simulink model "s_c856m4kfc"  (R2016b) (continued)
== system s_c856m4kfc == (continued)
      | disp(['Z^-', num2str(latency)]);
  we_delay2 : Subsystem  AncestorBlock=casper_library_delays/pipeline  mask(pipeline){latency=2}
      mask help:
      | An explicitly laid-out delay line to avoid mapping to SRL16s
      | for use in pipelining to help achieve timing closure.
      mask init:
      | pipeline_init(gcb,'latency',latency);
      settings: AttributesFormatString=Latency = %<latency>
      mask Display:
      | port_label('input', 1, 'd');
      | port_label('output', 1, 'q');
      | disp(['Z^-', num2str(latency)]);
  we_delay29 : Subsystem  AncestorBlock=casper_library_delays/pipeline  mask(pipeline){latency=2}
      mask help:
      | An explicitly laid-out delay line to avoid mapping to SRL16s
      | for use in pipelining to help achieve timing closure.
      mask init:
      | pipeline_init(gcb,'latency',latency);
      settings: AttributesFormatString=Latency = %<latency>
      mask Display:
      | port_label('input', 1, 'd');
      | port_label('output', 1, 'q');
      | disp(['Z^-', num2str(latency)]);
  we_delay37 : Subsystem  AncestorBlock=casper_library_delays/pipeline  mask(pipeline){latency=2}
      mask help:
      | An explicitly laid-out delay line to avoid mapping to SRL16s
      | for use in pipelining to help achieve timing closure.
      mask init:
      | pipeline_init(gcb,'latency',latency);
      settings: AttributesFormatString=Latency = %<latency>
      mask Display:
      | port_label('input', 1, 'd');
      | port_label('output', 1, 'q');
      | disp(['Z^-', num2str(latency)]);
  we_delay38 : Subsystem  AncestorBlock=casper_library_delays/pipeline  mask(pipeline){latency=2}
      mask help:
      | An explicitly laid-out delay line to avoid mapping to SRL16s
      | for use in pipelining to help achieve timing closure.
      mask init:
      | pipeline_init(gcb,'latency',latency);
      settings: AttributesFormatString=Latency = %<latency>
      mask Display:
      | port_label('input', 1, 'd');
      | port_label('output', 1, 'q');
      | disp(['Z^-', num2str(latency)]);
  we_delay39 : Subsystem  AncestorBlock=casper_library_delays/pipeline  mask(pipeline){latency=2}
      mask help:
      | An explicitly laid-out delay line to avoid mapping to SRL16s
      | for use in pipelining to help achieve timing closure.
      mask init:
      | pipeline_init(gcb,'latency',latency);
      settings: AttributesFormatString=Latency = %<latency>
      mask Display:
      | port_label('input', 1, 'd');
      | port_label('output', 1, 'q');
      | disp(['Z^-', num2str(latency)]);
  we_delay41 : Subsystem  AncestorBlock=casper_library_delays/pipeline  mask(pipeline){latency=2}
      mask help:
      | An explicitly laid-out delay line to avoid mapping to SRL16s
      | for use in pipelining to help achieve timing closure.
      mask init:
      | pipeline_init(gcb,'latency',latency);
      settings: AttributesFormatString=Latency = %<latency>
      mask Display:
      | port_label('input', 1, 'd');
      | port_label('output', 1, 'q');
      | disp(['Z^-', num2str(latency)]);
  we_delay47 : Subsystem  AncestorBlock=casper_library_delays/pipeline  mask(pipeline){latency=2}
      mask help:
      | An explicitly laid-out delay line to avoid mapping to SRL16s
      | for use in pipelining to help achieve timing closure.
      mask init:
      | pipeline_init(gcb,'latency',latency);
      settings: AttributesFormatString=Latency = %<latency>
      mask Display:
      | port_label('input', 1, 'd');
      | port_label('output', 1, 'q');
      | disp(['Z^-', num2str(latency)]);
  we_delay48 : Subsystem  AncestorBlock=casper_library_delays/pipeline  mask(pipeline){latency=2}
      mask help:
      | An explicitly laid-out delay line to avoid mapping to SRL16s
      | for use in pipelining to help achieve timing closure.
      mask init:
      | pipeline_init(gcb,'latency',latency);
      settings: AttributesFormatString=Latency = %<latency>
      mask Display:
      | port_label('input', 1, 'd');
      | port_label('output', 1, 'q');
      | disp(['Z^-', num2str(latency)]);
  we_delay49 : Subsystem  AncestorBlock=casper_library_delays/pipeline  mask(pipeline){latency=2}
      mask help:
      | An explicitly laid-out delay line to avoid mapping to SRL16s
      | for use in pipelining to help achieve timing closure.
      mask init:
      | pipeline_init(gcb,'latency',latency);
      settings: AttributesFormatString=Latency = %<latency>
      mask Display:
      | port_label('input', 1, 'd');
      | port_label('output', 1, 'q');
      | disp(['Z^-', num2str(latency)]);
  we_delay50 : Subsystem  AncestorBlock=casper_library_delays/pipeline  mask(pipeline){latency=2}
      mask help:
      | An explicitly laid-out delay line to avoid mapping to SRL16s
      | for use in pipelining to help achieve timing closure.
      mask init:
      | pipeline_init(gcb,'latency',latency);
      settings: AttributesFormatString=Latency = %<latency>
      mask Display:
      | port_label('input', 1, 'd');
      | port_label('output', 1, 'q');
      | disp(['Z^-', num2str(latency)]);
  we_delay7 : Subsystem  AncestorBlock=casper_library_delays/pipeline  mask(pipeline){latency=2}
      mask help:
      | An explicitly laid-out delay line to avoid mapping to SRL16s
      | for use in pipelining to help achieve timing closure.
      mask init:
      | pipeline_init(gcb,'latency',latency);
      settings: AttributesFormatString=Latency = %<latency>
      mask Display:
      | port_label('input', 1, 'd');
      | port_label('output', 1, 'q');
      | disp(['Z^-', num2str(latency)]);
  x_setup : Subsystem  Tag=xps:sw_reg  AncestorBlock=xps_library/software_register  mask(swreg){io_dir=From Processor, io_delay=0, sample_period=1, names=[f_per_x ip_per_x num_x snap_arm pktstart_mux pack_mux pktoffset_sel], bitwidths=[15 4 9 1 1 1 1], bin_pts=[0 2 0 0 0 0 0], arith_types=[0 0 0 2 0 2 0], sim_port=off, show_format=on}
      mask prompts: io_dir "I/O direction"; io_delay "I/O delay"; sample_period "Sample period"; names "Bitfield names [msb...lsb]"; bitwidths "Bitfield widths"; bin_pts "Bitfield binary pts"; arith_types "Bitfield types, ufix=0, fix=1, bool=2"; sim_port "Provide sim input/output?"; show_format "Print format string?"
      mask help: A 32-bit software-accessible register. Can be divided into bitfields of varying widths and types using the fields in the block mask.
      mask init:
      | swreg_init(gcb)
      settings: AttributesFormatString=7 inputs:uf15.0,uf4.2,uf9.0,b,uf1.0,b,uf1.0 = 32 bits
      mask TypeOptions io_dir: To Processor | From Processor
      mask Callback io_dir:
      | mask_enables = get_param(gcb, 'MaskEnables');
      | mask_names = get_param(gcb, 'MaskNames');
      | if strcmp(get_param(gcb, 'io_dir'), 'To Processor'),
      |     mask_enables{ismember(mask_names, 'sample_period')} = 'off';
      | else
      |     mask_enables{ismember(mask_names, 'sample_period')} = 'on';
      | end
      | set_param(gcb, 'MaskEnables', mask_enables);
      mask DialogControl ParameterTabVar0 Prompt: Setup
  xsg_core_config : Subsystem  Tag=xps:xsg  AncestorBlock=xps_library/XSG_core_config  mask(xsg core config){hw_sys=SKARAB:xc7vx690t, clk_src=sys_clk, clk_rate=238, sample_period=1, synthesis_tool=XST}
      mask prompts: hw_sys "Hardware Platform"; clk_src "User IP Clock source"; clk_rate "User IP Clock Rate (MHz)"; sample_period "Sample Period"; synthesis_tool "Synthesis Tool:"
      mask help:
      | The XSG Core Config block is used to configure the System Generator design
      | for the casper_xps toolflow. Settings here are used to configure the
      | Xilinx System Generator block parameters automatically, and control toolflow
      | script  execution. It needs to be at the top level of all designs being compiled
      | with the casper_xps toolflow.
      mask init:
      | xps_xsg_conf_mask;
      mask Help: eval('xlWeb([getenv(''MLIB_ROOT''), ''\doc\html\sys_xsg_core_config\sys_xsg_core_config.html''])')
      mask TypeOptions hw_sys: ROACH:sx95t | ROACH:lx110t | ROACH2:sx475t | SNAP:xc7k160t | KC705:xc7k325t | SKARAB:xc7vx690t
      mask Callback hw_sys: xps_xsg_conf_callback
      mask TypeOptions clk_src: sys_clk | aux_clk
      mask Callback clk_src: xps_xsg_conf_callback
      mask Callback clk_rate: xps_xsg_conf_callback
      mask TypeOptions synthesis_tool: Leonardo Spectrum | Synplify | Synplify Pro | XST
      mask Display: fprintf(['MSSGE\n', hw_sys]);
  tag scope: acc_scale=global, cnt_rst=global, ct_board_hmc_offset=global, ct_chans_per_board=global, ct_chans_per_x=global, ct_chans_per_x_hmc_par=global, ct_chans_per_x_hmc_par_recip=global, ct_chans_per_x_par=global, ct_chans_per_x_recip=global, ct_fake_data_error=global, ct_freq_gen_offset=global, ct_num_x_boards=global, ct_num_x_boards_recip=global, ct_num_xeng=global, ct_num_xeng_recip=global, ct_rst_err_latch=global, ct_tag_insert_sel=global, ct_tvg_en2=global, ct_tvg_en=global, ct_x_per_board=global, ct_x_per_board_recip=global, force_gbe_txen=global, obuf_read_gap=global, pfb_bypass=global, pktoffset_sel=global, pktstart_sel=global, sys_rst=global, ts_tvg_en=global, tvgtone_levels=global, tvgtone_p0=global, tvgtone_p1=global, up_timestep_error=global
  wires:
  Constant3/1 -> gbe0/8(rx_overrun_ack)
  Constant4/1 -> gbe0/7(rx_ack)
  [gbe_rst] -> pipeline99/1(d)
  unpack_and_reorder/5(recvd) -> ts_tvg/6(recvd_in)
  sync_gen1/2(dout) -> bus_expand6/1(bus_in)
  sync_gen1/3(dvalid) -> impulse_gen0/6(en), impulse_gen1/6(en)
  Slice4/1 -> pipeline16/1(d)
  pipeline16/1(q) -> local_time_lsw/1(out_timestamp_lsw)
  Slice16/1 -> Convert6/1
  pipeline47/1(q) -> local_time_msw/1(out_timestamp_msw)
  Convert6/1 -> pipeline47/1(d)
  [time80_48] -> Slice16/1, Slice4/1
  Slice12/1 -> [time80]
  impulse_gen0/3(dvalid) -> [valid80]
  impulse_gen0/1(sync_out) -> [sync80]
  impulse_gen0/2(dout) -> [d80_adc0]
  impulse_gen0/4(misco) -> [time80_48], Slice12/1
  [tvg_adc0_en] -> impulse_gen0/1(tvg_en)
  impulse_gen1/1(sync_out) -> Terminator6/1
  impulse_gen1/2(dout) -> [d80_adc1]
  impulse_gen1/3(dvalid) -> Terminator8/1
  impulse_gen1/4(misco) -> [dig_flags]
  [tvg_adc1_en] -> impulse_gen1/1(tvg_en)
  impulse0/1(in_offset) -> Slice7/1
  impulse0/2(in_amplitude) -> impulse_gen0/3(impulse_amplitude)
  impulse1/1(in_offset) -> Slice8/1
  impulse1/2(in_amplitude) -> impulse_gen1/3(impulse_amplitude)
  sync_gen1/1(sync) -> impulse_gen0/4(sync_in), impulse_gen1/4(sync_in)
  bus_expand6/1(msb_out2) -> impulse_gen0/5(din)
  bus_expand6/2(lsb_out1) -> impulse_gen1/5(din)
  sync_gen1/5(misco) -> impulse_gen1/7(misci)
  sync_gen1/4(time_out) -> impulse_gen0/7(misci)
  Constant31/1 -> Concat9/2
  [unpack_timestamp] -> Concat9/1
  [up_timestep_error] -> Logical15/1
  [sys_rst] -> Logical15/2
  Logical15/1 -> edge_detect17/1(in)
  edge_detect17/1(out) -> [sys_relock]
  Concat9/1 -> sync_gen1/1(time_in)
  [sys_relock] -> sync_gen1/5(relock)
  [unpack_dv] -> sync_gen1/2(en)
  [unpack_d160] -> sync_gen1/3(din)
  Slice7/1 -> impulse_gen0/2(impulse_offset)
  Slice8/1 -> impulse_gen1/2(impulse_offset)
  trig_time_msw/1(in_msw) -> pipeline28/1(d)
  trig_time_lsw/1(in_lsw) -> pipeline44/1(d)
  Concat13/1 -> Relational10/1
  pipeline28/1(q) -> Concat13/1
  pipeline44/1(q) -> Concat13/2
  [valid80] -> Counter/2
  [sync80] -> Counter/1
  Constant12/1 -> Relational7/2
  Counter/1 -> Relational7/1
  Relational7/1 -> Mux/3
  [adc_snap_arm] -> Logical14/2
  [time80_48] -> Relational10/2
  [adc_snap_trig_source] -> Mux/1
  edge_detect4/1(out) -> pipeline5/1(d)
  control/7(in_cnt_rst) -> edge_detect1/1(in)
  edge_detect1/1(out) -> pipeline14/1(d)
  control/3(in_gbe_rst) -> pipeline6/1(d)
  control/1(in_sys_rst) -> pipeline3/1(d)
  pipeline3/1(q) -> [sys_rst]
  pipeline5/1(q) -> [clr_status]
  pipeline6/1(q) -> [gbe_rst]
  pipeline7/1(q) -> [gbe_txen]
  pipeline13/1(q) -> [tvg_adc0_en]
  pipeline14/1(q) -> [cnt_rst]
  control/5(in_tvg_adc0) -> pipeline13/1(d)
  edge_detect2/1(out) -> pipeline38/1(d)
  pipeline38/1(q) -> [gbe_cnt_rst]
  control/8(in_gbe_cnt_rst) -> edge_detect2/1(in)
  control/4(in_gbe_txen) -> pipeline7/1(d)
  control/2(in_status_clr) -> edge_detect4/1(in)
  pipeline43/1(q) -> [adc_snap_arm]
  control/9(in_adc_snap_arm) -> pipeline43/1(d)
  control/10(in_adc_snap_trig_select) -> pipeline39/1(d)
  pipeline39/1(q) -> [adc_snap_trig_source]
  pfb/2(dout0) -> [pfb_d200_0]
  pfb/3(dout1) -> [pfb_d200_1]
  fft_shift/1(in_fft_shift) -> pipeline4/1(d)
  pfb/5(of) -> [pfb_of]
  pfb/4(dvalid) -> [pfb_d200_dv]
  pfb/1(sync_out) -> [pfb_d200_sync]
  tl_fd0/2(enable) -> phase_compensation0/5(load_value)
  tl_fd1/1(value) -> phase_compensation1/4(delay_value)
  tl_fd1/2(enable) -> phase_compensation1/5(load_value)
  tl_fd0/1(value) -> phase_compensation0/4(delay_value)
  [clr_status] -> tl_fd1/1(rst), tl_fd0/1(rst)
  phase_compensation0/1(sync_out) -> [fdfs_sync]
  phase_compensation1/1(sync_out) -> Terminator24/1
  phase_compensation0/2(dout) -> [fdfs_d144_0]
  phase_compensation1/2(dout) -> [fdfs_d144_1]
  phase_compensation0/3(dvalid) -> [fdfs_dv]
  phase_compensation1/3(dvalid) -> Terminator25/1
  [ld_val0] -> tl_fd0/3(load_value)
  [ld_val1] -> tl_fd1/3(load_value)
  bus_expand16/1(msb_out2) -> tl_fd0/5(din)
  tl_fd0/5(dvalid) -> phase_compensation0/3(en)
  tl_fd0/3(sync_out) -> phase_compensation0/1(sync_in)
  tl_fd0/4(dout) -> phase_compensation0/2(din)
  tl_fd1/4(dout) -> phase_compensation1/2(din)
  bus_expand16/2(lsb_out1) -> tl_fd1/5(din)
  Constant29/1 -> Concat2/2
  Concat2/1 -> tl_fd1/2(current_time), tl_fd0/2(current_time)
  [gbe_cnt_rst] -> pipeline31/1(d)
  pipeline31/1(q) -> gbe0/9(debug_rst)
  Counter2/1 -> pipeline29/1(d)
  Constant26/1 -> eq1/2(data_in)
  Constant11/1 -> eq1/3(we)
  Delay53/1 -> quant1/1(sync_in)
  Delay34/1 -> quant1/2(din)
  eq1/1(data_out) -> quant1/4(coeffs)
  Delay50/1 -> quant1/3(en)
  Counter4/1 -> pipeline27/1(d)
  Constant24/1 -> eq0/2(data_in)
  Constant25/1 -> eq0/3(we)
  Delay51/1 -> quant0/1(sync_in)
  Delay2/1 -> quant0/2(din)
  Delay52/1 -> quant0/3(en)
  eq0/1(data_out) -> quant0/4(coeffs)
  quant0/1(sync_out) -> [q_sync]
  quant0/2(dout) -> [q_d64_0]
  quant0/3(dvalid) -> [q_dv]
  quant0/4(of) -> [q_of0]
  quant1/2(dout) -> [q_d64_1]
  quant1/1(sync_out) -> Terminator19/1
  quant1/3(dvalid) -> Terminator21/1
  quant1/4(of) -> [q_of1]
  pipeline27/1(q) -> eq0/1(addr)
  pipeline29/1(q) -> eq1/1(addr)
  [fdfs_sync] -> Delay51/1, pipeline42/1(d)
  [fdfs_d144_0] -> Delay2/1
  [fdfs_dv] -> Delay52/1, pipeline41/1(d)
  pipeline41/1(q) -> Counter4/2
  pipeline42/1(q) -> Counter4/1
  pipeline45/1(q) -> Counter2/2
  [fdfs_sync] -> Delay53/1, pipeline48/1(d)
  [fdfs_d144_1] -> Delay34/1
  [fdfs_dv] -> Delay50/1, pipeline45/1(d)
  pipeline48/1(q) -> Counter2/1
  gbe0/13(rx_dest_ip) -> pipeline115/1(d)
  gbe0/14(rx_dest_port) -> pipeline116/1(d)
  [rx_dest_ip] -> pipeline/1(d)
  pipeline/1(q) -> rx_dest_ip/1(out_reg)
  gbe0/1(led_up) -> pipeline100/1(d)
  [forty_gbe_led_up] -> pipeline19/1(d)
  gbe0/2(led_rx) -> pipeline101/1(d)
  [forty_gbe_led_rx] -> pipeline20/1(d)
  gbe0/3(led_tx) -> pipeline102/1(d)
  [forty_gbe_led_tx] -> pipeline51/1(d)
  gbe0/4(tx_afull) -> pipeline103/1(d)
  gbe0/5(tx_overflow) -> pipeline104/1(d)
  gbe0/12(rx_overrun) -> pipeline114/1(d)
  pipeline19/1(q) -> gbe_status/1(out_led_up)
  pipeline20/1(q) -> gbe_status/2(out_led_rx)
  pipeline51/1(q) -> gbe_status/3(out_led_tx)
  gbe0/8(rx_source_ip) -> pipeline110/1(d)
  gbe0/9(rx_source_port) -> pipeline111/1(d)
  gbe0/6(rx_data) -> pipeline108/1(d)
  gbe0/10(rx_end_of_frame) -> pipeline112/1(d)
  gbe0/11(rx_bad_frame) -> pipeline113/1(d)
  pipeline80/1(q) -> [flag_sync]
  pipeline81/1(q) -> [flag_misc]
  pipeline82/1(q) -> [flag_dvalid]
  pipeline83/1(q) -> [flag_time]
  pipeline85/1(q) -> time_gen1/4(rd_sync_in)
  pipeline87/1(q) -> time_gen1/6(rd_misc_in)
  [s256_d] -> pipeline98/1(d)
  [s256_ip] -> pipeline97/1(d)
  [s256_port] -> pipeline96/1(d)
  Logical2/1 -> gbe0/6(tx_end_of_frame)
  [s256_v] -> Logical1/2
  [s256_eof] -> Logical2/2
  Delay1/1 -> Logical1/1, Logical2/1
  [s256_eof] -> Delay1/2
  pipeline96/1(q) -> gbe0/5(tx_dest_port)
  pipeline97/1(q) -> gbe0/4(tx_dest_ip)
  pipeline98/1(q) -> gbe0/2(tx_data)
  pipeline99/1(q) -> gbe0/1(rst)
  Logical4/1 -> Delay1/1
  pipeline100/1(q) -> [forty_gbe_led_up]
  pipeline101/1(q) -> [forty_gbe_led_rx]
  pipeline102/1(q) -> [forty_gbe_led_tx]
  pipeline103/1(q) -> [forty_gbe_tx_full]
  pipeline104/1(q) -> [forty_gbe_tx_of]
  pipeline108/1(q) -> [forty_gbe_rx_data]
  pipeline110/1(q) -> [forty_gbe_rx_src_ip]
  pipeline111/1(q) -> [forty_gbe_rx_src_port]
  pipeline112/1(q) -> [forty_gbe_rx_eof]
  pipeline113/1(q) -> [forty_gbe_rx_bad]
  pipeline114/1(q) -> [forty_gbe_rx_overrun]
  pipeline115/1(q) -> [rx_dest_ip]
  pipeline116/1(q) -> [rx_dest_port]
  [forty_gbe_rx_data] -> unpack_and_reorder/1(d256)
  [forty_gbe_rx_valid] -> unpack_and_reorder/2(dv256)
  [forty_gbe_rx_eof] -> unpack_and_reorder/3(eof256)
  unpack_and_reorder/2(d160) -> ts_tvg/3(data_in)
  unpack_and_reorder/3(dv) -> ts_tvg/4(dv_in)
  unpack_and_reorder/4(time) -> ts_tvg/5(time_in)
  unpack_and_reorder/1(sync) -> ts_tvg/2(sync_in)
  pipeline10/1(q) -> [unpack_sync]
  pipeline11/1(q) -> [unpack_d160]
  pipeline12/1(q) -> [unpack_dv]
  pipeline15/1(q) -> [unpack_timestamp]
  [clr_status] -> Subsystem5/1(rst)
  Delay/1 -> pipeline85/1(d)  "gapsync"
  Delay3/1 -> pipeline86/1(d)  "gapdata"
  Mux1/1 -> pipeline87/1(d)  "gapvalid"
  tl_fd0/7(misco) -> Terminator2/1
  tl_fd0/6(time) -> Terminator3/1
  tl_fd1/6(time) -> Terminator4/1
  tl_fd1/3(sync_out) -> phase_compensation1/1(sync_in)
  tl_fd1/5(dvalid) -> phase_compensation1/3(en)
  tl_fd1/7(misco) -> Terminator5/1
  Constant18/1 -> tl_fd0/7(misci)
  Constant19/1 -> tl_fd1/7(misci)
  Constant1/1 -> sync_gen1/4(misci)
  time_gen/1(rd_sync_out) -> tl_fd1/4(sync_in), tl_fd0/4(sync_in)
  time_gen/2(rd_dvalid) -> tl_fd1/6(en), tl_fd0/6(en)
  time_gen/3(rd_val_out) -> Concat2/1
  time_gen/4(rd_misc_out) -> bus_expand16/1(bus_in)
  [sync80] -> time_gen/1(wr_sync_in)
  [valid80] -> time_gen/2(wr_en)
  [pfb_sync] -> time_gen/4(rd_sync_in)
  [pfb_dv] -> time_gen/5(rd_en)
  [pfb_d144_1] -> Concat1/2
  [pfb_d144_0] -> Concat1/1
  Concat1/1 -> time_gen/6(rd_misc_in)
  [time80] -> Slice2/1
  pipeline86/1(q) -> time_gen1/5(rd_en)
  time_gen1/1(rd_sync_out) -> pipeline80/1(d)
  time_gen1/4(rd_misc_out) -> pipeline81/1(d)
  time_gen1/2(rd_dvalid) -> pipeline82/1(d)
  time_gen1/3(rd_val_out) -> pipeline83/1(d)
  [time80] -> time_gen/3(wr_val_in)
  Slice2/1 -> time_gen1/3(wr_val_in)
  pipeline4/1(q) -> [fft_shift]
  Logical1/1 -> gbe0/3(tx_valid)
  pipeline59/1(q) -> [ct_tvg_en]
  control/11(in_ct_tvg_en) -> pipeline59/1(d)
  pipeline52/1(q) -> [up_dbg_rst]
  control/12(in_unpack_debug_reset) -> pipeline52/1(d)
  [clr_status] -> Subsystem3/1(rst)
  Slice/1 -> Subsystem3/2(in0)
  [pfb_of] -> Slice/1, Slice6/1
  Slice6/1 -> Subsystem3/3(in1)
  [pfb_sync] -> Subsystem3/4(in2)
  Subsystem3/3(out2) -> pfb_status/3(out_sync_cnt)
  Subsystem5/1(out0) -> quant_status/1(out_pol0_or_cnt)
  Relational/1 -> Subsystem5/2(in0)
  Constant10/1 -> Relational/2
  [q_of0] -> Relational/1
  Relational3/1 -> Subsystem5/3(in1)
  [q_of1] -> Relational3/1
  Constant13/1 -> Relational3/2
  Subsystem5/2(out1) -> quant_status/2(out_pol1_or_cnt)
  Subsystem3/1(out0) -> pfb_status/1(out_pol0_or_err_cnt)
  Subsystem3/2(out1) -> pfb_status/2(out_pol1_or_err_cnt)
  [ct_tvg_en2] -> Mux1/1
  Delay17/1 -> Delay5/1
  Delay6/1 -> Mux1/2
  Delay7/1 -> tvg/1(sync), Delay/1
  Delay18/1 -> Delay7/1
  Delay16/1 -> Delay6/1
  Delay5/1 -> tvg/2(dv), Delay3/1
  tvg/1(data256) -> Mux1/3
  [q_dv] -> qt_block_check/1(dv)
  [cnt_rst] -> Subsystem12/1(rst)
  Subsystem12/1(out0) -> qt_dv_err/1(out_dvblock_err_cnt)
  qt_block_check/1(error) -> Subsystem12/2(in0)
  [cd_bypass] -> Terminator28/1
  [cd_bypass] -> Terminator26/1
  [delay_sync] -> [pfbin_sync]
  [delay_dv] -> [pfbin_dv]
  [cd_bypass] -> Terminator22/1
  [delay_d0] -> [pfbin_d0]
  [cd_bypass] -> Terminator18/1
  [delay_d1] -> [pfbin_d1]
  control/13(in_cd_bypass) -> pipeline131/1(d)
  pipeline131/1(q) -> [cd_bypass]
  Concat22/1 -> [ld_val0]
  Concat3/1 -> [ld_val1]
  phase0/1(in_initial) -> Reinterpret2/1
  delta_delay0/1(in_delta) -> Reinterpret1/1
  phase0/2(in_delta) -> Reinterpret3/1
  Reinterpret8/1 -> Concat22/1
  Reinterpret1/1 -> Concat22/2
  Reinterpret2/1 -> Concat22/3
  Reinterpret3/1 -> Concat22/4
  phase1/1(in_initial) -> Reinterpret5/1
  delta_delay1/1(in_delta) -> Reinterpret4/1
  phase1/2(in_delta) -> Reinterpret6/1
  Reinterpret4/1 -> Concat3/2
  Reinterpret5/1 -> Concat3/3
  Reinterpret6/1 -> Concat3/4
  Reinterpret7/1 -> Concat3/1
  delay0/1(in_initial) -> Reinterpret8/1
  delay1/1(in_initial) -> Reinterpret7/1
  [clr_status] -> Subsystem2/1(rst)
  [cd_error] -> Subsystem2/2(in0)
  [flag_dvalid] -> Subsystem13/1(In1)
  Subsystem13/1(Out1) -> pack_in_dv_rate/1(out_dv_rate)
  [s256_v] -> Subsystem11/1(In1)
  Subsystem11/1(Out1) -> pack_out_dv_rate/1(out_dv_rate)
  pipeline121/1(q) -> Convert/1
  pipeline124/1(q) -> [ip_per_x]
  pipeline125/1(q) -> [num_x]
  x_setup/1(in_f_per_x) -> pipeline121/1(d)
  x_setup/2(in_ip_per_x) -> pipeline124/1(d)
  x_setup/3(in_num_x) -> pipeline125/1(d)
  pipeline120/1(q) -> [pack_snap_arm]
  x_setup/4(in_snap_arm) -> pipeline120/1(d)
  pipeline130/1(q) -> [pktstart_sel]
  x_setup/5(in_pktstart_mux) -> pipeline130/1(d)
  [s256_v] -> pack_block_check/1(dv)
  [cnt_rst] -> Subsystem14/1(rst)
  Subsystem14/1(out0) -> pack_dv_err/1(out_dvblock_err_cnt)
  pack_block_check/1(error) -> [pack_dv_err], Subsystem14/2(in0)
  pipeline149/1(q) -> [pack_mux]
  x_setup/6(in_pack_mux) -> pipeline149/1(d)
  tl_cd0_control0/3(in_load_time_msw) -> Concat6/1
  tl_cd0_control/1(in_load_time_lsw) -> Concat6/2
  Constant42/1 -> tl_cd0_control/1(sim_1)
  Constant40/1 -> tl_cd0_control0/3(sim_3)
  Constant41/1 -> tl_cd0_control0/2(sim_2)
  Constant43/1 -> Delay12/1
  Delay12/1 -> tl_cd0_control0/1(sim_1)
  Concat6/1 -> pipeline136/1(d)
  tl_cd0_control0/2(in_load_immediate) -> pipeline135/1(d)
  tl_cd0_control0/1(in_arm) -> pipeline134/1(d)
  tl_cd1_control0/3(in_load_time_msw) -> Concat8/1
  tl_cd1_control/1(in_load_time_lsw) -> Concat8/2
  Constant47/1 -> tl_cd1_control/1(sim_1)
  Constant45/1 -> tl_cd1_control0/3(sim_3)
  Constant46/1 -> tl_cd1_control0/2(sim_2)
  Constant48/1 -> Delay13/1
  Delay13/1 -> tl_cd1_control0/1(sim_1)
  Concat8/1 -> pipeline143/1(d)
  tl_cd1_control0/2(in_load_immediate) -> pipeline138/1(d)
  tl_cd1_control0/1(in_arm) -> pipeline137/1(d)
  [ld_val0] -> pipeline73/1(d)
  [clr_status] -> pipeline25/1(d), pipeline75/1(d)
  tl_cd0/6(time) -> [time80_tl_cd0]
  Constant6/1 -> Concat15/2
  Concat15/1 -> pipeline24/1(d), Gateway Out7/1, pipeline74/1(d)
  [time80] -> Concat15/1
  pipeline75/1(q) -> tl_cd0/1(rst)
  pipeline74/1(q) -> tl_cd0/2(current_time)
  pipeline73/1(q) -> tl_cd0/3(load_value)
  pipeline72/1(q) -> tl_cd0/4(sync_in)
  pipeline71/1(q) -> tl_cd0/5(din)
  pipeline76/1(q) -> tl_cd0/6(en)
  [sync80] -> pipeline36/1(d), pipeline72/1(d)
  [valid80] -> pipeline34/1(d), pipeline76/1(d)
  Constant16/1 -> tl_cd0/7(misci)
  tl_cd0/7(misco) -> Terminator/1
  tl_cd0/3(sync_out) -> [sync_tl_cd0]
  tl_cd0/5(dvalid) -> [dvalid_tl_cd0]
  tl_cd0/4(dout) -> [dout_tl_cd0]
  tl_cd0/2(enable) -> [en_tl_cd0]
  tl_cd0/1(value) -> [delay_tl_cd0]
  pipeline92/1(q) -> tl_cd0/10(load_time)
  pipeline91/1(q) -> tl_cd0/9(load_immediate)
  pipeline90/1(q) -> tl_cd0/8(arm)
  [tl_cd0_arm] -> pipeline90/1(d)
  [tl_cd0_load_immediate] -> pipeline91/1(d)
  [tl_cd0_load_time] -> pipeline92/1(d)
  Gateway Out7/1 -> To Workspace7/1
  [d80_adc0] -> pipeline71/1(d)
  [dvalid_tl_cd0] -> Delay22/1
  [sync_tl_cd0] -> Delay21/1
  [en_tl_cd0] -> Delay24/1
  [delay_tl_cd0] -> Delay23/1
  Concat5/1 -> time_gen_cd/6(rd_misc_in)
  time_gen_cd/4(rd_misc_out) -> bus_expand2/1(bus_in)
  [sync_tl_cd0] -> time_gen_cd/1(wr_sync_in)
  [dvalid_tl_cd0] -> time_gen_cd/2(wr_en)
  [hmc_sync_p0] -> time_gen_cd/4(rd_sync_in)
  [hmc_dvalid] -> time_gen_cd/5(rd_en)
  time_gen_cd/1(rd_sync_out) -> [tg_rd_sync]
  time_gen_cd/2(rd_dvalid) -> [tg_rd_dvalid]
  [dvalid_tl_bs0] -> wb_prog_delay/4(en)
  [delay_tl_bs0] -> wb_prog_delay/2(delay_val)
  [delay_tl_sync0] -> wb_prog_delay/1(sync)
  [en_tl_bs0] -> wb_prog_delay/5(load_val)
  wb_prog_delay/2(data_out) -> [delay_d0]
  wb_prog_delay/3(dvalid) -> [delay_dv]
  [time80_tl_cd0] -> Slice9/1
  Slice9/1 -> time_gen_cd/3(wr_val_in)
  Constant35/1 -> Concat7/2
  time_gen_cd/3(rd_val_out) -> Concat7/1
  Concat7/1 -> [tg_rd_time_out]
  wb_prog_delay/1(sync_out) -> [delay_sync]
  pipeline77/1(q) -> tl_cd_bs0/1(rst)
  pipeline1/1(q) -> tl_cd_bs0/2(current_time)
  pipeline78/1(q) -> tl_cd_bs0/3(load_value)
  pipeline84/1(q) -> tl_cd_bs0/4(sync_in)
  pipeline88/1(q) -> tl_cd_bs0/5(din)
  pipeline89/1(q) -> tl_cd_bs0/6(en)
  [clr_status] -> pipeline77/1(d)
  [tg_rd_time_out] -> pipeline1/1(d)
  [ld_val0] -> pipeline78/1(d)
  [tg_rd_sync] -> pipeline84/1(d)
  [tg_rd_dvalid] -> pipeline89/1(d)
  Constant34/1 -> tl_cd_bs0/7(misci)
  tl_cd_bs0/7(misco) -> Terminator12/1
  tl_cd_bs0/4(dout) -> [data_tl_bs0]
  tl_cd_bs0/6(time) -> Terminator13/1
  tl_cd_bs0/5(dvalid) -> [dvalid_tl_bs0]
  tl_cd_bs0/1(value) -> [delay_tl_bs0]
  tl_cd_bs0/3(sync_out) -> [delay_tl_sync0]
  tl_cd_bs0/2(enable) -> [en_tl_bs0]
  pipeline21/1(q) -> tl_cd_bs0/8(arm)
  pipeline22/1(q) -> tl_cd_bs0/9(load_immediate)
  pipeline23/1(q) -> tl_cd_bs0/10(load_time)
  [tl_cd0_arm] -> pipeline21/1(d)
  [tl_cd0_load_immediate] -> pipeline22/1(d)
  [tl_cd0_load_time] -> pipeline23/1(d)
  [data_tl_bs0] -> wb_prog_delay/3(din)
  [tg_data_p0] -> pipeline88/1(d)
  pipeline25/1(q) -> tl_cd1/1(rst)
  [ld_val1] -> pipeline26/1(d)
  pipeline24/1(q) -> tl_cd1/2(current_time)
  pipeline26/1(q) -> tl_cd1/3(load_value)
  pipeline36/1(q) -> tl_cd1/4(sync_in)
  pipeline33/1(q) -> tl_cd1/5(din)
  pipeline34/1(q) -> tl_cd1/6(en)
  pipeline37/1(q) -> tl_cd1/9(load_immediate)
  pipeline35/1(q) -> tl_cd1/8(arm)
  pipeline40/1(q) -> tl_cd1/10(load_time)
  [tl_cd1_arm] -> pipeline35/1(d)
  [tl_cd1_load_immediate] -> pipeline37/1(d)
  [tl_cd1_load_time] -> pipeline40/1(d)
  Constant17/1 -> tl_cd1/7(misci)
  tl_cd1/1(value) -> [delay_tl_cd1]
  tl_cd1/2(enable) -> [en_tl_cd1]
  tl_cd1/3(sync_out) -> [sync_tl_cd1]
  tl_cd1/4(dout) -> [dout_tl_cd1]
  tl_cd1/5(dvalid) -> [dvalid_tl_cd1]
  tl_cd1/6(time) -> [time80_tl_cd1]
  tl_cd1/7(misco) -> Terminator1/1
  [delay_tl_cd1] -> Delay27/1
  [en_tl_cd1] -> Delay28/1
  [sync_tl_cd1] -> Delay25/1
  [dvalid_tl_cd1] -> Delay26/1
  pipeline54/1(q) -> tl_cd_bs1/1(rst)
  pipeline53/1(q) -> tl_cd_bs1/2(current_time)
  pipeline60/1(q) -> tl_cd_bs1/3(load_value)
  pipeline61/1(q) -> tl_cd_bs1/4(sync_in)
  pipeline67/1(q) -> tl_cd_bs1/5(din)
  pipeline68/1(q) -> tl_cd_bs1/6(en)
  [clr_status] -> pipeline54/1(d)
  [tg_rd_time_out] -> pipeline53/1(d)
  [ld_val1] -> pipeline60/1(d)
  [tg_rd_sync] -> pipeline61/1(d)
  [tg_rd_dvalid] -> pipeline68/1(d)
  [tg_data_p1] -> pipeline67/1(d)
  Constant36/1 -> tl_cd_bs1/7(misci)
  pipeline156/1(q) -> tl_cd_bs1/8(arm)
  pipeline69/1(q) -> tl_cd_bs1/9(load_immediate)
  pipeline70/1(q) -> tl_cd_bs1/10(load_time)
  [tl_cd1_load_time] -> pipeline70/1(d)
  [tl_cd1_load_immediate] -> pipeline69/1(d)
  [tl_cd1_arm] -> pipeline156/1(d)
  tl_cd_bs1/7(misco) -> Terminator10/1
  tl_cd_bs1/4(dout) -> [data_tl_bs1]
  tl_cd_bs1/6(time) -> Terminator7/1
  tl_cd_bs1/5(dvalid) -> [dvalid_tl_bs1]
  tl_cd_bs1/1(value) -> [delay_tl_bs1]
  tl_cd_bs1/3(sync_out) -> [delay_tl_sync1]
  tl_cd_bs1/2(enable) -> [en_tl_bs1]
  [delay_tl_sync1] -> wb_prog_delay1/1(sync)
  [delay_tl_bs1] -> wb_prog_delay1/2(delay_val)
  [en_tl_bs1] -> wb_prog_delay1/5(load_val)
  [data_tl_bs1] -> wb_prog_delay1/3(din)
  [dvalid_tl_bs1] -> wb_prog_delay1/4(en)
  wb_prog_delay1/2(data_out) -> [delay_d1]
  wb_prog_delay1/1(sync_out) -> Terminator16/1
  wb_prog_delay1/3(dvalid) -> Terminator11/1
  [d80_adc1] -> pipeline33/1(d)
  Subsystem7/1(per_sec) -> reorder_status1/1(out_dv_per_sec)
  [upg_reo_time] -> Subsystem1/3(time)
  [upg_reo_recv] -> Subsystem1/4(recv)
  [upg_reo_dv] -> Subsystem7/1(dv), Subsystem1/2(dv)
  [cnt_rst] -> Subsystem1/1(rst)
  Subsystem1/7(time_change) -> [reo_time_change]
  Logical5/1 -> Subsystem1/7(overflow_err)
  [upg_of80_p1] -> Logical5/2
  [upg_of80_p0] -> Logical5/1
  Subsystem1/8(timestep_err) -> [up_timestep_error]
  [upg_reo_disc] -> Subsystem1/5(discard_err)
  [upg_reo_relock] -> Subsystem1/6(relock_err)
  Subsystem1/1(timestep_err_cnt) -> reorder_status/1(out_timestep_err_cnt)
  Subsystem1/2(recv_err_cnt) -> reorder_status/2(out_receive_err_cnt)
  Subsystem1/3(discard_err_cnt) -> reorder_status/3(out_discard_cnt)
  Subsystem1/4(relock_err_cnt) -> reorder_status/4(out_relock_err_cnt)
  Subsystem1/5(overflow_err_cnt) -> reorder_status/5(out_overflow_err_cnt)
  Subsystem1/6(dv_cnt) -> reorder_status/6(out_dv_cnt)
  pipeline93/1(q) -> [tvg_adc1_en]
  control/6(in_tvg_adc1) -> pipeline93/1(d)
  pipeline57/1(q) -> bus_expand7/1(bus_in)
  [valid80] -> Slice5/1
  Slice14/1 -> pipeline162/1(d)
  [time80_48] -> Slice14/1
  pipeline162/1(q) -> snap_adc0/11(extra_timestamp)
  Slice5/1 -> pipeline161/1(d)
  [d80_adc0] -> pipeline57/1(d)
  bus_expand7/4(out5) -> snap_adc0/4(in_p0_d3)
  bus_expand7/5(out4) -> snap_adc0/5(in_p0_d4)
  bus_expand7/1(msb_out8) -> snap_adc0/1(in_p0_d0)
  bus_expand7/2(out7) -> snap_adc0/2(in_p0_d1)
  bus_expand7/3(out6) -> snap_adc0/3(in_p0_d2)
  bus_expand7/6(out3) -> snap_adc0/6(in_p0_d5)
  bus_expand7/7(out2) -> snap_adc0/7(in_p0_d6)
  bus_expand7/8(lsb_out1) -> snap_adc0/8(in_p0_d7)
  pipeline161/1(q) -> snap_adc0/9(we)
  gbe0/7(rx_valid) -> pipeline62/1(d)
  pipeline62/1(q) -> [forty_gbe_rx_valid]
  Counter1/1 -> pipeline165/1(d)
  pipeline165/1(q) -> sync80_count/1(out_reg)
  pipeline65/1(q) -> Counter1/1
  pipeline109/1(q) -> Counter1/2
  [cnt_rst] -> edge_detect9/1(in)
  [sync80] -> edge_detect10/1(in)
  edge_detect9/1(out) -> pipeline65/1(d)
  edge_detect10/1(out) -> pipeline109/1(d)
  Convert/1 -> [f_per_x]
  [sync80] -> Terminator27/1
  [d80_adc0] -> Terminator20/1
  [d80_adc1] -> Terminator17/1
  [ct_d256] -> Delay16/1
  [ct_dv] -> Delay17/1
  [ct_sync] -> Delay18/1
  [valid80] -> Terminator23/1
  [hmc_data_p0] -> Concat5/1
  [hmc_data_p1] -> Concat5/2
  bus_expand2/1(msb_out2) -> [tg_data_p0]
  bus_expand2/2(lsb_out1) -> [tg_data_p1]
  control/14(in_gbe_stopmux) -> pipeline9/1(d)
  pipeline9/1(q) -> [gbe_stopmux]
  x_setup/7(in_pktoffset_sel) -> pipeline63/1(d)
  pipeline63/1(q) -> [pktoffset_sel]
  control/15(in_pfb_bypass) -> pipeline66/1(d)
  pipeline66/1(q) -> [pfb_bypass]
  Delay21/1 -> cd_hmc/1(sync_in)
  Delay22/1 -> cd_hmc/3(en)
  Delay23/1 -> cd_hmc/4(delay_val)
  Delay24/1 -> cd_hmc/5(load_val)
  [dout_tl_cd0] -> Delay29/1
  pipeline134/1(q) -> [tl_cd0_arm]
  pipeline135/1(q) -> [tl_cd0_load_immediate]
  pipeline136/1(q) -> [tl_cd0_load_time]
  pipeline138/1(q) -> [tl_cd1_load_immediate]
  pipeline137/1(q) -> [tl_cd1_arm]
  pipeline143/1(q) -> [tl_cd1_load_time]
  Delay29/1 -> cd_hmc/2(din)
  [dout_tl_cd1] -> Delay30/1
  ts_tvg/1(sync) -> pipeline10/1(d)
  ts_tvg/2(d160) -> pipeline11/1(d)
  ts_tvg/3(dv) -> pipeline12/1(d)
  ts_tvg/4(time) -> pipeline15/1(d)
  ts_tvg/5(recvd) -> Terminator9/1
  control/16(in_ts_tvg_en) -> pipeline148/1(d)
  pipeline148/1(q) -> [ts_tvg_en]
  [ts_tvg_en] -> ts_tvg/1(tvg_en)
  [pfbin_d0] -> pfb/3(din0)
  [fft_shift] -> pfb/1(shift)
  [pfbin_sync] -> pfb/2(sync_in)
  [pfbin_d1] -> pfb/4(din1)
  [pfbin_dv] -> pfb/5(en)
  Delay25/1 -> cd_hmc/6(sync_in1)
  Delay30/1 -> cd_hmc/7(din1)
  Delay26/1 -> cd_hmc/8(en1)
  Delay27/1 -> cd_hmc/9(delay_val1)
  Delay28/1 -> cd_hmc/10(load_val1)
  Subsystem2/1(out0) -> cd_status/1(out_err_cnt)
  [pack_sync_time] -> Subsystem15/1(time)
  [flag_sync] -> Subsystem15/2(In2)
  [flag_dvalid] -> Subsystem15/3(In3)
  Subsystem15/1(Out1) -> pack_sync_time_msw/1(out_reg)
  Subsystem15/2(Out2) -> pack_sync_time_lsw/1(out_reg)
  iptx_base/1(in_reg) -> pipeline105/1(d)
  pipeline105/1(q) -> [tx_ip_base]
  tx_metadata/1(in_porttx) -> pipeline106/1(d)
  tx_metadata/2(in_board_id) -> pipeline107/1(d)
  pipeline106/1(q) -> [tx_port]
  pipeline107/1(q) -> [tx_board_id]
  [hmc_dvalid] -> Subsystem2/3(in1)
  Subsystem2/2(out1) -> cd_status/2(out_dv_cnt)
  tvg_tone_levels/2(in_p0i) -> bus_create/2(in2)
  tvg_tone_levels/3(in_p1r) -> bus_create/3(in3)
  tvg_tone_levels/4(in_p1i) -> bus_create/4(in4)
  tvg_tone_levels/1(in_p0r) -> bus_create/1(in1)
  tvg_tone_targets/1(in_p0) -> Slice18/1
  tvg_tone_targets/2(in_p1) -> Slice19/1
  Constant51/1 -> tvg_tone_targets/1(sim_1)
  Constant52/1 -> tvg_tone_targets/2(sim_2)
  Constant37/1 -> tvg_tone_levels/4(sim_4)
  Constant2/1 -> tvg_tone_levels/1(sim_1)
  Constant27/1 -> tvg_tone_levels/3(sim_3)
  Constant5/1 -> tvg_tone_levels/2(sim_2)
  bus_create/1(bus_out) -> [tvgtone_levels]
  pipeline17/1(q) -> [outfreq_stopmux]
  ct_debug/6(in_outfreq_stopmux) -> pipeline17/1(d)
  pipeline18/1(q) -> [out_stopmux]
  ct_debug/5(in_out_stopmux) -> pipeline18/1(d)
  pipeline55/1(q) -> [wrrd_stopmux]
  ct_debug/3(in_wrrd_stopmux) -> pipeline55/1(d)
  pipeline56/1(q) -> [in_stopmux]
  ct_debug/1(in_in_stopmux) -> pipeline56/1(d)
  pipeline122/1(q) -> [ct_snap_arm]
  pipeline147/1(q) -> edge_detect5/1
  ct_debug/8(in_snap_trig) -> pipeline147/1(d)
  ct_debug/7(in_snap_arm) -> pipeline122/1(d)
  edge_detect5/1 -> [ct_snap_trig]
  ct_debug/2(in_in_trigmux) -> pipeline150/1(d)
  pipeline150/1(q) -> [in_trigmux]
  ct_debug/4(in_obuf_stopmux) -> pipeline167/1(d)
  pipeline167/1(q) -> [obuf_stopmux]
  ct_debug/9(in_snap_wesel) -> pipeline8/1(d)
  pipeline8/1(q) -> [ct_snap_wesel]
  pipeline157/1(q) -> [ct_snap_trigsel]
  ct_debug/10(in_snap_trigsel) -> pipeline157/1(d)
  pipeline166/1(q) -> [ct_snap_circsel]
  ct_debug/11(in_snap_circsel) -> pipeline166/1(d)
  [ct_dv] -> Subsystem8/1(In1)
  Subsystem8/1(Out1) -> ct_out_dv_rate/1(out_reg)
  [cnt_rst] -> Subsystem10/1(rst)
  [obuf_ct_addr_err_g] -> Subsystem10/2(in0)
  Subsystem10/1(out0) -> ct_status2/1(out_addr_err0_cnt)
  Subsystem10/2(out1) -> ct_status2/2(out_sync_cnt)
  [ct_sync] -> Subsystem10/3(in1)
  [ct_pktstart] -> we_delay1/1(d)
  [ct_dv] -> we_delay2/1(d)
  we_delay1/1(q) -> pktlen_check/1(pkt_start)
  we_delay2/1(q) -> dv_block_check/1(dv), pktlen_check/2(dv)
  pktlen_check/1(error) -> we_delay7/1(d)
  dv_block_check/1(error) -> we_delay29/1(d)
  [cnt_rst] -> Subsystem6/1(rst)
  we_delay7/1(q) -> [ct_pktlen_err], Subsystem6/2(in0)
  we_delay29/1(q) -> [ct_dvblk_err], Subsystem6/3(in1)
  Subsystem6/1(out0) -> ct_dv_err/1(out_pktlen_err_cnt)
  Subsystem6/2(out1) -> ct_dv_err/2(out_dvblock_err_cnt)
  [cnt_rst] -> Subsystem9/1(rst)
  [ct_bank_error_g0] -> Subsystem9/2(in0)
  [ct_en_sync_error_g0] -> Subsystem9/3(in1)
  [ct_d256] -> Slice43/1
  Slice43/1 -> Relational21/2
  [ct_pktfreq] -> Relational21/1
  Relational21/1 -> Logical16/2
  [ct_dv] -> Delay19/1
  Delay19/1 -> Logical16/1
  Logical16/1 -> [freq_sync_error]
  [cnt_rst] -> Subsystem16/1(rst)
  [cnt_rst] -> Subsystem18/1(rst)
  [sync_hmc_in0] -> Subsystem18/2(in0)
  [q_sync] -> Subsystem19/5(in3)
  [pfb_sync] -> Subsystem19/3(in1)
  [fdfs_sync] -> Subsystem19/4(in2)
  [sync80] -> Subsystem19/2(in0)
  [cnt_rst] -> Subsystem19/1(rst)
  Subsystem19/1(out0) -> sync_ctrs1/1(out_sync80)
  Subsystem19/2(out1) -> sync_ctrs1/2(out_pfb)
  Subsystem19/3(out2) -> sync_ctrs1/3(out_fdfs)
  Subsystem19/4(out3) -> sync_ctrs1/4(out_quant)
  [freq_sync_error] -> Subsystem16/3(in1)
  [rd_go_err_g] -> Subsystem16/2(in0)
  Subsystem16/1(out0) -> ct_status3/1(out_out_go_err)
  Subsystem16/2(out1) -> ct_status3/2(out_freq_sync_err)
  [cnt_rst] -> Subsystem20/1(rst)
  [ct_en_sync_error_g1] -> Subsystem9/5(in3)
  [ct_bank_error_g1] -> Subsystem9/4(in2)
  Subsystem9/2(out1) -> ct_status0/2(out_err_en_sync0)
  Subsystem9/4(out3) -> ct_status0/4(out_err_en_sync1)
  Subsystem9/1(out0) -> ct_status0/1(out_err_bank0)
  Subsystem9/3(out2) -> ct_status0/3(out_err_bank1)
  [hmc_err_rdrdy_g0] -> Subsystem20/3(in1)
  [hmc_err_wrrdy_g0] -> Subsystem20/2(in0)
  Subsystem20/2(out1) -> ct_status1/2(out_err_wrrdy0)
  Subsystem20/1(out0) -> ct_status1/1(out_err_rdrdy0)
  Subsystem20/4(out3) -> ct_status1/4(out_err_rdrdy1)
  Subsystem20/6(out5) -> ct_status1/6(out_wr_ctr1)
  Subsystem20/5(out4) -> ct_status1/5(out_err_wrrdy1)
  Subsystem20/3(out2) -> ct_status1/3(out_wr_ctr0)
  [wr_en_final_g0] -> Subsystem20/4(in2)
  [hmc_err_wrrdy_g1] -> Subsystem20/5(in3)
  [hmc_err_rdrdy_g1] -> Subsystem20/6(in4)
  [wr_en_final_g1] -> Subsystem20/7(in5)
  [cnt_rst] -> Subsystem21/1(rst)
  [hmc_postokay_g0] -> Inverter/1
  [hmc_init_done_g0] -> Inverter3/1
  [hmc_init_done_g1] -> Inverter5/1
  [hmc_postokay_g1] -> Inverter4/1
  Subsystem21/2(out1) -> ct_status4/2(out_init_err0)
  Subsystem21/4(out3) -> ct_status4/4(out_init_err1)
  Subsystem21/3(out2) -> ct_status4/3(out_post_err1)
  Subsystem21/1(out0) -> ct_status4/1(out_post_err0)
  Inverter/1 -> Subsystem21/2(in0)
  Inverter3/1 -> Subsystem21/3(in1)
  Inverter4/1 -> Subsystem21/4(in2)
  Inverter5/1 -> Subsystem21/5(in3)
  [cnt_rst] -> Subsystem22/1(rst)
  [hmc_dv_clash_g] -> Subsystem22/2(in0)
  [hmc_dv_fixed_clash_g] -> Subsystem22/3(in1)
  [hmc_fifo_full_g] -> Subsystem22/4(in2)
  Subsystem22/3(out2) -> ct_status5/3(out_fifo_full_err)
  Subsystem22/1(out0) -> ct_status5/1(out_dv_clash)
  Subsystem22/2(out1) -> ct_status5/2(out_dv_fixed_clash)
  [sync_hmc_in1] -> Subsystem18/3(in1)
  [sync_obuf_in] -> Subsystem18/4(in2)
  [sync_obuf_out] -> Subsystem18/5(in3)
  Subsystem18/1(out0) -> sync_ctrs2/1(out_hmc_in0)
  Subsystem18/2(out1) -> sync_ctrs2/2(out_hmc_in1)
  Subsystem18/3(out2) -> sync_ctrs2/3(out_hmc_out)
  Subsystem18/4(out3) -> sync_ctrs2/4(out_obuf_out)
  [cthmc_sync_err_g0] -> Subsystem16/4(in2)
  Subsystem16/3(out2) -> ct_status3/3(out_hmc_sync_error)
  pipeline185/1(q) -> pipeline181/1(d)
  pipeline177/1(q) -> pipeline179/1(d)
  pipeline179/1(q) -> pipeline180/1(d)
  pipeline180/1(q) -> pipeline185/1(d)
  pipeline181/1(q) -> [flag_pktstart]
  pipeline182/1(q) -> pipeline183/1(d)
  pipeline183/1(q) -> pipeline184/1(d)
  pipeline187/1(q) -> pipeline186/1(d)
  pipeline186/1(q) -> [flag_pktfreq]
  pipeline184/1(q) -> pipeline187/1(d)
  pipeline168/1(q) -> pipeline169/1(d)
  pipeline169/1(q) -> pipeline170/1(d)
  pipeline170/1(q) -> pipeline171/1(d)
  pipeline171/1(q) -> pipeline172/1(d)
  pipeline172/1(q) -> [flag_pktxindex]
  pipeline173/1(q) -> pipeline174/1(d)
  pipeline174/1(q) -> pipeline175/1(d)
  pipeline175/1(q) -> pipeline176/1(d)
  pipeline176/1(q) -> pipeline178/1(d)
  pipeline178/1(q) -> [flag_pktxoffset]
  [ct_pkt_x_index] -> Delay15/1
  [ct_pkt_x_offset] -> Delay20/1
  [ct_pktfreq] -> Delay14/1
  [ct_pktstart] -> Delay4/1
  Delay4/1 -> pipeline177/1(d)
  Delay14/1 -> pipeline182/1(d)
  Delay15/1 -> pipeline168/1(d)
  Delay20/1 -> pipeline173/1(d)
  [flag_sync] -> packetiser/1(sync_in)
  [flag_dvalid] -> packetiser/2(en)
  [flag_pktstart] -> packetiser/5(pkt_start)
  [flag_pktxindex] -> Slice20/1
  [flag_pktxoffset] -> packetiser/7(pkt_x_offset)
  [tx_ip_base] -> packetiser/9(base_ip)
  [tx_port] -> packetiser/10(base_port)
  Constant28/1 -> concat1/2
  [flag_time] -> concat1/1
  [flag_misc] -> packetiser/3(din)
  concat1/1 -> [pack_sync_time], packetiser/4(timestamp)
  pipeline117/1(q) -> [s256_d]
  pipeline118/1(q) -> [s256_v]
  pipeline129/1(q) -> [s256_eof]
  pipeline139/1(q) -> [s256_of]
  pipeline140/1(q) -> [s256_ip]
  pipeline141/1(q) -> [s256_port]
  pipeline142/1(q) -> [s256_sync]
  packetiser/1(dout) -> pipeline117/1(d)
  packetiser/2(dvalid) -> pipeline118/1(d)
  packetiser/3(eof) -> pipeline129/1(d)
  packetiser/4(overflow) -> pipeline139/1(d)
  packetiser/5(destination_ip) -> pipeline140/1(d)
  packetiser/6(destination_port) -> pipeline141/1(d)
  packetiser/7(sync) -> pipeline142/1(d)
  [flag_pktfreq] -> pipeline64/1(d)
  pipeline151/1(q) -> [s256_pktfreq]
  pipeline64/1(q) -> pipeline151/1(d)
  [flag_pktxindex] -> pipeline94/1(d)
  pipeline94/1(q) -> pipeline79/1(d)
  pipeline79/1(q) -> [s256_pktxindex]
  [flag_pktxoffset] -> pipeline152/1(d)
  pipeline152/1(q) -> pipeline95/1(d)
  pipeline95/1(q) -> [s256_pktxoffset]
  [tx_board_id] -> packetiser/8(board_id)
  [flag_pktstart] -> pipeline153/1(d)
  pipeline153/1(q) -> pipeline58/1(d)
  pipeline58/1(q) -> [s256_pktstart]
  we_delay49/1(q) -> [ct_tag_insert_sel]
  edge_detect3/1 -> [ct_rst_err_latch]
  we_delay47/1(q) -> edge_detect3/1
  we_delay48/1(q) -> [ct_fake_data_error]
  we_delay13/1(q) -> [ct_tvg_en]
  we_delay50/1(q) -> [ct_tvg_en2]
  Constant7/1 -> ct_control0/5(sim_5)
  Constant21/1 -> ct_control0/4(sim_4)
  Constant20/1 -> ct_control0/3(sim_3)
  Constant22/1 -> ct_control0/2(sim_2)
  Constant15/1 -> ct_control0/1(sim_1)
  ct_control0/1(in_tvg_en) -> we_delay13/1(d)
  ct_control0/2(in_tvg_en2) -> we_delay50/1(d)
  ct_control0/3(in_rst_err_latch) -> we_delay47/1(d)
  ct_control0/4(in_fake_error) -> we_delay48/1(d)
  ct_control0/5(in_tag_insert) -> we_delay49/1(d)
  we_delay126/1(q) -> [obuf_read_gap]
  ct_control0/6(in_obuf_read_gap) -> we_delay126/1(d)
  Constant30/1 -> ct_control0/6(sim_6)
  we_delay41/1(q) -> [ct_num_xeng]
  we_delay112/1(q) -> [ct_num_xeng_recip]  "NUM_X_RECIP"
  ct_control1/2(in_num_x_recip) -> we_delay112/1(d)
  ct_control1/1(in_num_x) -> we_delay41/1(d)
  we_delay114/1(q) -> [ct_x_per_board]
  ct_control1/3(in_x_per_board) -> we_delay114/1(d)
  we_delay39/1(q) -> [ct_chans_per_x], Slice3/1  "PER_X"
  we_delay113/1(q) -> [ct_chans_per_board]
  ct_control2/1(in_chans_per_x) -> we_delay39/1(d)
  ct_control2/2(in_chans_per_board) -> we_delay113/1(d)
  Slice3/1 -> Slice39/1, [ct_chans_per_x_par]  "PARPAR"
  we_delay115/1(q) -> [ct_x_per_board_recip]
  ct_control1/4(in_x_per_board_recip) -> we_delay115/1(d)
  we_delay38/1(q) -> [ct_num_x_boards]
  ct_control3/1(in_num_x_boards) -> we_delay38/1(d)
  we_delay37/1(q) -> [ct_num_x_boards_recip]
  ct_control3/2(in_num_x_boards_recip) -> we_delay37/1(d)
  ct_control3/3(in_chans_per_x_recip) -> we_delay124/1(d)
  we_delay124/1(q) -> Reinterpret40/1, [ct_chans_per_x_recip]
  Slice39/1 -> [ct_chans_per_x_hmc_par]  "per_x_hmc"
  Reinterpret40/1 -> [ct_chans_per_x_hmc_par_recip]
  Constant49/1 -> ct_control1/1(sim_1)
  Constant53/1 -> ct_control1/2(sim_2)
  Constant33/1 -> ct_control1/3(sim_3)
  Constant23/1 -> ct_control2/1(sim_1)
  Constant50/1 -> ct_control2/2(sim_2)
  Constant54/1 -> ct_control1/4(sim_4)
  Constant32/1 -> ct_control3/1(sim_1)
  Constant14/1 -> ct_control3/2(sim_2)
  Constant44/1 -> ct_control3/3(sim_3)
  Slice18/1 -> [tvgtone_p0]
  Slice19/1 -> [tvgtone_p1]
  [sync80] -> time_gen1/1(wr_sync_in)
  [valid80] -> time_gen1/2(wr_en)
  [time80_48] -> Subsystem/1(time)
  [sync80] -> Subsystem/2(sync)
  [valid80] -> Subsystem/3(dv)
  Subsystem/1(msw) -> sync_time_msw/1(out_reg)
  Subsystem/2(ls32) -> sync_time_lsw/1(out_reg)
  [q_dv] -> Subsystem17/1(In1)
  Subsystem17/1(Out1) -> ct_in_dv_rate/1(out_reg)
  [pfb_dv] -> Subsystem24/1(In1)
  Subsystem24/1(Out1) -> pfb_out_dv_rate/1(out_reg)
  [pfbin_dv] -> Subsystem23/1(In1)
  Subsystem23/1(Out1) -> pfb_in_dv_rate/1(out_reg)
  [hmc_dvalid] -> Subsystem26/1(In1)
  Subsystem26/1(Out1) -> cd_out_dv_rate/1(out_reg)
  [dvalid_tl_cd0] -> Subsystem25/1(In1)
  Subsystem25/1(Out1) -> cd_in_dv_rate/1(out_reg)
  Slice36/1 -> we_delay116/1(d)
  Constant38/1 -> ct_control4/1(sim_1)
  ct_control4/1(in_ct_board_offset) -> Slice36/1
  ct_control5/1(in_ct_freq_gen_offset) -> we_delay117/1(d)
  Constant39/1 -> ct_control5/1(sim_1)
  we_delay116/1(q) -> [ct_board_hmc_offset]
  we_delay117/1(q) -> [ct_freq_gen_offset]
  Slice20/1 -> packetiser/6(pkt_x_index)
  sync_mangle/1(sync_out) -> [hmc_sync_p0]
  sync_mangle/2(dout0) -> [hmc_data_p0]
  sync_mangle/4(dvalid) -> [hmc_dvalid]
  cd_hmc/1(sync_out) -> sync_mangle/1(sync_in)
  cd_hmc/2(dout0) -> sync_mangle/2(din)
  cd_hmc/4(dvalid) -> sync_mangle/4(en)
  cd_hmc/5(hmc_error) -> [cd_error]
  cd_hmc/3(dout1) -> sync_mangle/3(din1)
  sync_mangle/3(dout1) -> [hmc_data_p1]
  [obuf_proc_err_miss_g] -> Subsystem22/5(in3)
  Subsystem22/4(out3) -> ct_status5/4(out_proc_err_miss)
  control/17(in_force_gbe_txen) -> pipeline2/1(d)
  pipeline2/1(q) -> [force_gbe_txen]
  [sys_relock] -> Register/2
  [s256_sync] -> Register/3, Register/1
  Register/1 -> Logical6/1
  [gbe_txen] -> Logical4/2
  [force_gbe_txen] -> Logical6/2
  Logical6/1 -> Logical4/1
  [flag_pktfreq] -> Subsystem27/3(value)
  [cnt_rst] -> Subsystem27/1(rst)
  [flag_dvalid] -> Subsystem27/2(en)
  Subsystem27/1(current) -> flag_pktfreq_stats/1(out_current)
  Subsystem27/3(min) -> flag_pktfreq_stats/3(out_min)
  Subsystem27/2(max) -> flag_pktfreq_stats/2(out_max)
  [flag_pktxindex] -> Subsystem28/3(value)
  [cnt_rst] -> Subsystem28/1(rst)
  [flag_dvalid] -> Subsystem28/2(en)
  Subsystem28/1(current) -> flag_pktxindex_stats/1(out_current)
  Subsystem28/3(min) -> flag_pktxindex_stats/3(out_min)
  Subsystem28/2(max) -> flag_pktxindex_stats/2(out_max)
  [flag_pktxoffset] -> Subsystem29/3(value)
  [cnt_rst] -> Subsystem29/1(rst)
  [flag_dvalid] -> Subsystem29/2(en)
  Subsystem29/1(current) -> flag_pktxoffset_stats/1(out_current)
  Subsystem29/3(min) -> flag_pktxoffset_stats/3(out_min)
  Subsystem29/2(max) -> flag_pktxoffset_stats/2(out_max)
  [flag_time] -> Subsystem30/3(value)
  [cnt_rst] -> Subsystem30/1(rst)
  [flag_pktstart] -> Subsystem30/2(en)
  Subsystem30/1(current) -> flag_time_stats/1(out_current)
  Subsystem30/3(min) -> flag_time_stats/3(out_min)
  Subsystem30/2(max) -> flag_time_stats/2(out_max)
  [s256_ip] -> Subsystem31/3(value)
  [cnt_rst] -> Subsystem31/1(rst)
  [s256_v] -> Subsystem31/2(en)
  Subsystem31/1(current) -> pack_ip_stats/1(out_current)
  Subsystem31/3(min) -> pack_ip_stats/3(out_min)
  Subsystem31/2(max) -> pack_ip_stats/2(out_max)
  [cnt_rst] -> Subsystem32/1(rst)
  Subsystem32/1(current) -> pack_eof_stats/1(out_current)
  Subsystem32/3(min) -> pack_eof_stats/3(out_min)
  Subsystem32/2(max) -> pack_eof_stats/2(out_max)
  [s256_v] -> Subsystem32/2(en)
  [s256_eof] -> Reinterpret/1
  [upg_sperr_magic] -> Subsystem33/2(in0)
  [upg_sperr_len] -> Subsystem33/5(in3)
  [upg_sperr_hdr] -> Subsystem33/3(in1)
  [upg_sperr_pad] -> Subsystem33/4(in2)
  Subsystem33/5(out4) -> unpack_status/5(out_time_err_cnt)
  [upg_sperr_time] -> Subsystem33/6(in4)
  Subsystem33/4(out3) -> unpack_status/4(out_pkt_len_err_cnt)
  Subsystem33/3(out2) -> unpack_status/3(out_pad_err_cnt)
  Subsystem33/2(out1) -> unpack_status/2(out_header_err_cnt)
  Subsystem33/1(out0) -> unpack_status/1(out_magic_err_cnt)
  [cnt_rst] -> Subsystem4/1(rst), Subsystem33/1(rst)
  Logical3/1 -> Subsystem4/2(in0)
  Subsystem4/1(out0) -> unpack_status1/1(out_pkt_cnt)
  [upg_sp_dv] -> Logical3/2
  [upg_sp_eof] -> Logical3/1
  Reinterpret/1 -> Subsystem32/3(value)
  [flag_time] -> pipeline195/1(d)
  pipeline195/1(q) -> pipeline196/1(d)
  pipeline196/1(q) -> [s256_time]
  Mux/1 -> Logical14/1
  Relational10/1 -> Logical12/1
  [valid80] -> Logical12/2
  Logical12/1 -> Mux/2
  Logical14/1 -> snap_adc0/10(trig)
  [q_sync] -> hmc_ct/1(in_sync)
  hmc_ct/4(pkt_start) -> [ct_pktstart]
  hmc_ct/1(out_sync) -> [ct_sync]
  hmc_ct/2(out_dv) -> [ct_dv]
  hmc_ct/3(out_d256) -> [ct_d256]
  hmc_ct/5(freq_id) -> [ct_pktfreq]
  [q_dv] -> hmc_ct/2(in_dv)
  Concat4/1 -> hmc_ct/3(in_d128), [q_d128]
  hmc_ct/6(x_index) -> [ct_pkt_x_index]
  hmc_ct/7(x_pkt_index) -> [ct_pkt_x_offset]
  [q_d64_0] -> Concat4/1
  [q_d64_1] -> Concat4/2
  [pfb_d200_0] -> float_to_fix_0/1(d_in)
  [acc_scale] -> float_to_fix_0/2(Scale)
  Constant8/1 -> acc_scale/1(sim_1)
  acc_scale/1(in_reg) -> pipeline46/1(d)
  pipeline46/1(q) -> [acc_scale]
  float_to_fix_0/1(d_out) -> pipeline49/1(d)
  [pfb_d200_dv] -> float_to_fix_1/3(sync_in), float_to_fix_0/3(sync_in)
  [pfb_d200_sync] -> float_to_fix_1/4(dvalid_in), float_to_fix_0/4(dvalid_in)
  [pfb_d200_1] -> float_to_fix_1/1(d_in)
  [acc_scale] -> float_to_fix_1/2(Scale)
  float_to_fix_1/1(d_out) -> pipeline123/1(d)
  float_to_fix_1/2(sync_out) -> Terminator14/1
  float_to_fix_1/3(dvalid_out) -> Terminator15/1
  float_to_fix_0/3(dvalid_out) -> pipeline119/1(d)
  float_to_fix_0/2(sync_out) -> pipeline50/1(d)
  pipeline49/1(q) -> [pfb_d144_0]
  pipeline50/1(q) -> [pfb_sync]
  pipeline119/1(q) -> [pfb_dv]
  pipeline123/1(q) -> [pfb_d144_1]
